simulink model "TosAeb"  (R2021a)
settings: Solver=FixedStepDiscrete  StartTime=0.0  StopTime=inf  FixedStep=0.01  RelTol=1e-3
undefined symbols (not defined in the file or in its folder): ACCModSts, AccSts1, AEB_ObjectMovingOrientation, AEB_ObjectMovingState, CN_BTCActive, CN_CurrentGearR, CN_CurrentGearR_DHT, CN_PTCActive, CN_VDCActive, CN_VSC_EspOff, CN_VSC_FCW_AEB_Error, CN_VSC_FCW_AEB_NoDispay, CN_VSC_FCW_AEB_PerfmDgdtn, CN_VSC_FCW_AEB_TempUnAvlbl, CN_VSC_VehStandstill, CNaccelAvoidMax, CNaccelAvoidMin, CNbrakePedalPress, CNcomfortStrLeftAccelMax, CNcomfortStrLeftAccelMin, CNcomfortStrRightAccelMax, CNcomfortStrRightAccelMin, CNDrvDoorOpen, CNDrvModeEco, CNDrvModeNorm, CNDrvModeOther, CNDrvModeSport, CNDrvSBRreminder, CNNull, CNobjInvalidID, CNPassDoorOpen, CNPassSBRreminder, CNPEBSAccelNegInf, CNPEBSAccelNull, CNPEBSTimeInf, CNPEBSTimeNull, CNSTOPMAX, CNSTOPNULL, CNsubjWidth, CNSwtOFF, CNSwtON, CNTTCMAX, CNTTCNULL, CNVehConfigAEBCycNone, CNVehConfigAEBNone, CNVehConfigAEBPedNone, CNVehConfigFCWNone, CNVehConfigJANone, CollisionSeverity, FALSE, FCWSnvty, JACycObjCalcInfoDefaultParams, JAPedObjCalcInfoDefaultParams, JAVehObjCalcInfoDefaultParams, latCycObjCalcInfoDefaultParams, latPedObjCalcInfoDefaultParams, longObjCalcInfoDefaultParams, ModSts, movingStatus, ObjectType ... (+1112)
libraries: simulink x3031, AEBSFunctionLib x50, Ta_Library x33, AEBSStateMachineLib x23, Mylibrary x12, simulink_extras x7, ObjSelect_lib x3, dspstat3 x2
(part 64 of 102)

== system TosAeb/FcwAebJa_Step_sys/ALG_AEBSystem/ALG_AEBController/AEB_LongTgtInfoCalc/LongObjCalcInfoCalc/AEBS_LongCollisionAssesApp/LatCollisionAvoidCalc/LatAvoidFlg4WarningJudge/accelLatAvoidThesMO/AccelLatAvoidThesCalcHmiLate ==
  subjVel : Inport
  Action Port : ActionPort  ActionPortLabel=default:
  accelLatAvoidThesLookupTable : Lookup_n-D  NumberOfTableDimensions=1  BreakpointsForDimension1=P_AEB_LongCA_LatAvoidAccelThesWarnHmiLateMOX  BeginIndexSearchUsingPreviousIndexResult=on  Table=P_AEB_LongCA_LatAvoidAccelThesWarnHmiLateMOY  ExtrapMethod=Clip  InternalRulePriority=Speed  RndMeth=Simplest  InputSameDT=on
  accelLatAvoidThes : Outport
  wires:
  subjVel/1 -> accelLatAvoidThesLookupTable/1
  accelLatAvoidThesLookupTable/1 -> accelLatAvoidThes/1

== system TosAeb/FcwAebJa_Step_sys/ALG_AEBSystem/ALG_AEBController/AEB_LongTgtInfoCalc/LongObjCalcInfoCalc/AEBS_LongCollisionAssesApp/LatCollisionAvoidCalc/LatAvoidFlg4WarningJudge/accelLatAvoidThesMO/AccelLatAvoidThesCalcHmiMid ==
  subjVel : Inport
  Action Port : ActionPort  ActionPortLabel=case [ HMISettingMiddle ]:
  accelLatAvoidThesLookupTable : Lookup_n-D  NumberOfTableDimensions=1  BreakpointsForDimension1=P_AEB_LongCA_LatAvoidAccelThesWarnHmiMidMOX  BeginIndexSearchUsingPreviousIndexResult=on  Table=P_AEB_LongCA_LatAvoidAccelThesWarnHmiMidMOY  ExtrapMethod=Clip  InternalRulePriority=Speed  RndMeth=Simplest  InputSameDT=on
  accelLatAvoidThes : Outport
  wires:
  subjVel/1 -> accelLatAvoidThesLookupTable/1
  accelLatAvoidThesLookupTable/1 -> accelLatAvoidThes/1

== system TosAeb/FcwAebJa_Step_sys/ALG_AEBSystem/ALG_AEBController/AEB_LongTgtInfoCalc/LongObjCalcInfoCalc/AEBS_LongCollisionAssesApp/LatCollisionAvoidCalc/LatAvoidFlg4WarningJudge/accelLatAvoidThesSO/AccelLatAvoidThesCalcHmiEarly ==
  subjVel : Inport
  Action Port : ActionPort  ActionPortLabel=case [ HMISettingEarly ]:
  accelLatAvoidThesLookupTable : Lookup_n-D  NumberOfTableDimensions=1  BreakpointsForDimension1=P_AEB_LongCA_LatAvoidAccelThesWarnHmiEarlySOX  BeginIndexSearchUsingPreviousIndexResult=on  Table=P_AEB_LongCA_LatAvoidAccelThesWarnHmiEarlySOY  ExtrapMethod=Clip  InternalRulePriority=Speed  RndMeth=Simplest  InputSameDT=on
  accelLatAvoidThes : Outport
  wires:
  accelLatAvoidThesLookupTable/1 -> accelLatAvoidThes/1
  subjVel/1 -> accelLatAvoidThesLookupTable/1

== system TosAeb/FcwAebJa_Step_sys/ALG_AEBSystem/ALG_AEBController/AEB_LongTgtInfoCalc/LongObjCalcInfoCalc/AEBS_LongCollisionAssesApp/LatCollisionAvoidCalc/LatAvoidFlg4WarningJudge/accelLatAvoidThesSO/AccelLatAvoidThesCalcHmiLate ==
  subjVel : Inport
  Action Port : ActionPort  ActionPortLabel=default:
  accelLatAvoidThesLookupTable : Lookup_n-D  NumberOfTableDimensions=1  BreakpointsForDimension1=P_AEB_LongCA_LatAvoidAccelThesWarnHmiLateSOX  BeginIndexSearchUsingPreviousIndexResult=on  Table=P_AEB_LongCA_LatAvoidAccelThesWarnHmiLateSOY  ExtrapMethod=Clip  InternalRulePriority=Speed  RndMeth=Simplest  InputSameDT=on
  accelLatAvoidThes : Outport
  wires:
  accelLatAvoidThesLookupTable/1 -> accelLatAvoidThes/1
  subjVel/1 -> accelLatAvoidThesLookupTable/1

== system TosAeb/FcwAebJa_Step_sys/ALG_AEBSystem/ALG_AEBController/AEB_LongTgtInfoCalc/LongObjCalcInfoCalc/AEBS_LongCollisionAssesApp/LatCollisionAvoidCalc/LatAvoidFlg4WarningJudge/accelLatAvoidThesSO/AccelLatAvoidThesCalcHmiMid ==
  subjVel : Inport
  Action Port : ActionPort  ActionPortLabel=case [ HMISettingMiddle ]:
  accelLatAvoidThesLookupTable : Lookup_n-D  NumberOfTableDimensions=1  BreakpointsForDimension1=P_AEB_LongCA_LatAvoidAccelThesWarnHmiMidSOX  BeginIndexSearchUsingPreviousIndexResult=on  Table=P_AEB_LongCA_LatAvoidAccelThesWarnHmiMidSOY  ExtrapMethod=Clip  InternalRulePriority=Speed  RndMeth=Simplest  InputSameDT=on
  accelLatAvoidThes : Outport
  wires:
  accelLatAvoidThesLookupTable/1 -> accelLatAvoidThes/1
  subjVel/1 -> accelLatAvoidThesLookupTable/1

== system TosAeb/FcwAebJa_Step_sys/ALG_AEBSystem/ALG_AEBController/AEB_LongTgtInfoCalc/LongObjCalcInfoCalc/AEBS_LongCollisionAssesApp/LongCollisionAvoidAssess/HighLvlBrkCalc/HighLvlBrkCalc4Brake/HighLvlBrkFlgJudge ==
  accelAvoidHighLvlBrk : Inport
  subjVel : Inport  Port=2
  Compare To Constant : simulink/Logic and Bit Operations/Compare To Constant  relop===  const=FALSE  OutDataTypeStr=boolean
  HighLvlBrkAccelAvoidThes4Brk : Lookup_n-D  NumberOfTableDimensions=1  BreakpointsForDimension1=P_AEB_LongCA_HighLvlBrkAccelAvoidThes4BrkX  Table=P_AEB_LongCA_HighLvlBrkAccelAvoidThes4BrkY  ExtrapMethod=Clip  InternalRulePriority=Speed  RndMeth=Simplest  InputSameDT=on
  P_LongCA_HighLvlBrkAccelAvoidThes : Constant  Commented=on  Value=P_AEB_LongCA_HighLvlBrkAccelAvoidThes4Warn
  Relational Operator : RelationalOperator  Operator=<=  OutDataTypeStr=boolean  RndMeth=Simplest
  highLvlBrkFlg : Outport
  wires:
  HighLvlBrkAccelAvoidThes4Brk/1 -> Relational Operator/2  "AEB_LongCA_HighLvlBrkAccelAvoidThes4Brk"
  accelAvoidHighLvlBrk/1 -> Relational Operator/1
  Relational Operator/1 -> Compare To Constant/1
  Compare To Constant/1 -> highLvlBrkFlg/1
  subjVel/1 -> HighLvlBrkAccelAvoidThes4Brk/1

== system TosAeb/FcwAebJa_Step_sys/ALG_AEBSystem/ALG_AEBController/AEB_LongTgtInfoCalc/LongObjCalcInfoCalc/AEBS_LongCollisionAssesApp/LongCollisionAvoidAssess/HighLvlBrkCalc/HighLvlBrkCalc4Brake/HighLvlBrkFlgJudge1 ==
  accelAvoidHighLvlBrk : Inport
  Logical Operator : Logic  Operator=NOT  AllPortsSameDT=off  OutDataTypeStr=boolean
  P_LongCA_HighLvlBrkAccelAvoidThes : Constant  Value=P_AEB_LongCA_HighLvlBrkAccelAvoidThes4Brake
  Relational Operator : RelationalOperator  Operator=<=  OutDataTypeStr=boolean  RndMeth=Simplest
  highLvlBrkFlg : Outport
  wires:
  P_LongCA_HighLvlBrkAccelAvoidThes/1 -> Relational Operator/2
  Relational Operator/1 -> Logical Operator/1
  accelAvoidHighLvlBrk/1 -> Relational Operator/1
  Logical Operator/1 -> highLvlBrkFlg/1

== system TosAeb/FcwAebJa_Step_sys/ALG_AEBSystem/ALG_AEBController/AEB_LongTgtInfoCalc/LongObjCalcInfoCalc/AEBS_LongCollisionAssesApp/LongCollisionAvoidAssess/HighLvlBrkCalc/HighLvlBrkCalc4Brake/accelAvoidHighLvlBrkSituation1 ==
  vx : Inport
  axAbsObj : Inport  Port=2
  vxAbsObj : Inport  Port=3
  subjVel : Inport  Port=4
  tObjStop : Inport  Port=5
  dxEgoBrake : Inport  Port=6
  function : TriggerPort  TriggerType=function-call  VariantControl=Variant
  accelAvoidLowLvlBrkCalc : Subsystem  TreatAsAtomicUnit=on
  accelAvoidLowLvlBrkCalc2 : Subsystem  Commented=on  TreatAsAtomicUnit=on
  accelAvoidHighLvlBrk : Outport
  wires:
  vx/1 -> accelAvoidLowLvlBrkCalc/1(vx)
  axAbsObj/1 -> accelAvoidLowLvlBrkCalc/2(axAbsObj)
  vxAbsObj/1 -> accelAvoidLowLvlBrkCalc/3(vxAbsObj)
  subjVel/1 -> accelAvoidLowLvlBrkCalc/4(subjVel)
  tObjStop/1 -> accelAvoidLowLvlBrkCalc/5(tObjStop)
  dxEgoBrake/1 -> accelAvoidLowLvlBrkCalc/6(dxEgoBrake)
  accelAvoidLowLvlBrkCalc/1(accelAvoidLowLvlBrk) -> accelAvoidHighLvlBrk/1

== system TosAeb/FcwAebJa_Step_sys/ALG_AEBSystem/ALG_AEBController/AEB_LongTgtInfoCalc/LongObjCalcInfoCalc/AEBS_LongCollisionAssesApp/LongCollisionAvoidAssess/HighLvlBrkCalc/HighLvlBrkCalc4Brake/accelAvoidHighLvlBrkSituation2 ==
  tReaction : Inport
  TTC : Inport  Port=2
  dxFrontBumper : Inport  Port=3
  vx : Inport  Port=4
  ax : Inport  Port=5
  subjLongAccel : Inport  Port=6
  subjVel : Inport  Port=7
  axAbsObj : Inport  Port=8
  function : TriggerPort  TriggerType=function-call  VariantControl=Variant
  CollisionJuge : Subsystem
  CollisonOcurred : Subsystem  TreatAsAtomicUnit=on
  If : If  IfExpression=u1
  Merge : Merge
  accelAvoidHighLvlBrkCalc : Subsystem  TreatAsAtomicUnit=on
  accelAvoidHighLvlBrk : Outport
  wires:
  tReaction/1 -> CollisionJuge/1(tReaction), accelAvoidHighLvlBrkCalc/3(tReaction)
  CollisionJuge/1(collisonFlg) -> If/1
  TTC/1 -> CollisionJuge/2(TTC)
  If/1 -> CollisonOcurred/Action
  If/2 -> accelAvoidHighLvlBrkCalc/Action
  vx/1 -> accelAvoidHighLvlBrkCalc/2(vx)
  ax/1 -> accelAvoidHighLvlBrkCalc/4(ax)
  subjLongAccel/1 -> accelAvoidHighLvlBrkCalc/5(subjLongAccel)
  subjVel/1 -> accelAvoidHighLvlBrkCalc/6(subjVel)
  axAbsObj/1 -> accelAvoidHighLvlBrkCalc/7(axAbsObj)
  CollisonOcurred/1(accelAvoidHighLvlBrk) -> Merge/1
  accelAvoidHighLvlBrkCalc/1(accelAvoidHighLvlBrk) -> Merge/2
  Merge/1 -> accelAvoidHighLvlBrk/1
  dxFrontBumper/1 -> accelAvoidHighLvlBrkCalc/1(dxFrontBumper)

== system TosAeb/FcwAebJa_Step_sys/ALG_AEBSystem/ALG_AEBController/AEB_LongTgtInfoCalc/LongObjCalcInfoCalc/AEBS_LongCollisionAssesApp/LongCollisionAvoidAssess/HighLvlBrkCalc/HighLvlBrkCalc4Brake/accelAvoidHighLvlBrkSituation3 ==
  note: if tRaction > TTC
      | 	CollisionOcurred
      | else
      | 	caculate AccelAvoid
  tReaction : Inport
  TTC : Inport  Port=2
  subjLongAccel : Inport  Port=3
  subjVel : Inport  Port=4
  axAbsObj : Inport  Port=5
  vxAbsObj : Inport  Port=6
  dxFrontBumper : Inport  Port=7
  function : TriggerPort  TriggerType=function-call  VariantControl=Variant
  CollisionJuge : Subsystem
  If : If  IfExpression=u1
  If Action Subsystem : Subsystem  TreatAsAtomicUnit=on
  If Action Subsystem1 : Subsystem  TreatAsAtomicUnit=on
  Merge : Merge
  accelAvoidHighLvlBrk : Outport
  wires:
  CollisionJuge/1(collisonFlg) -> If/1
  TTC/1 -> CollisionJuge/2(TTC)
  tReaction/1 -> CollisionJuge/1(tReaction), If Action Subsystem1/2(tReaction)
  If/1 -> If Action Subsystem/Action
  If/2 -> If Action Subsystem1/Action
  subjLongAccel/1 -> If Action Subsystem1/1(subjLongAccel)
  subjVel/1 -> If Action Subsystem1/3(subjVel)
  axAbsObj/1 -> If Action Subsystem1/4(axAbsObj)
  vxAbsObj/1 -> If Action Subsystem1/5(vxAbsObj)
  dxFrontBumper/1 -> If Action Subsystem1/6(dxFrontBumper)
  If Action Subsystem/1(accelAvoidHighLvlBrk) -> Merge/1
  If Action Subsystem1/1(accelAvoidHighLvlBrk) -> Merge/2
  Merge/1 -> accelAvoidHighLvlBrk/1

== system TosAeb/FcwAebJa_Step_sys/ALG_AEBSystem/ALG_AEBController/AEB_LongTgtInfoCalc/LongObjCalcInfoCalc/AEBS_LongCollisionAssesApp/LongCollisionAvoidAssess/HighLvlBrkCalc/HighLvlBrkCalc4Brake/accelAvoidHighLvlBrkSituation4 ==
  tReaction : Inport
  TTC : Inport  Port=2
  function : TriggerPort  TriggerType=function-call  VariantControl=Variant
  accelAvoidHighLvlBrkCalc : Subsystem
  accelAvoidHighLvlBrk : Outport
  wires:
  tReaction/1 -> accelAvoidHighLvlBrkCalc/1(tReaction)
  TTC/1 -> accelAvoidHighLvlBrkCalc/2(TTC)
  accelAvoidHighLvlBrkCalc/1(accelAvoidHighLvlBrk) -> accelAvoidHighLvlBrk/1

== system TosAeb/FcwAebJa_Step_sys/ALG_AEBSystem/ALG_AEBController/AEB_LongTgtInfoCalc/LongObjCalcInfoCalc/AEBS_LongCollisionAssesApp/LongCollisionAvoidAssess/HighLvlBrkCalc/HighLvlBrkCalc4Brake/accelAvoidHighLvlBrkSituation5 ==
  tEgoStop : Inport
  tObjStop : Inport  Port=2
  tReaction : Inport  Port=3
  TTC : Inport  Port=4
  subjLongAccel : Inport  Port=5
  subjVel : Inport  Port=6
  axAbsObj : Inport  Port=7
  dxFrontBumper : Inport  Port=8
  vxAbsObj : Inport  Port=9
  function : TriggerPort  TriggerType=function-call  VariantControl=Variant
  If : If  NumInputs=2  IfExpression=u1 <= u2
  If Action Subsystem : Subsystem  TreatAsAtomicUnit=on
  If Action Subsystem1 : Subsystem  TreatAsAtomicUnit=on
  Merge : Merge
  accelAvoidHighLvlBrk : Outport
  wires:
  [tEgoStop] -> If Action Subsystem1/4(tEgoStop)
  vxAbsObj/1 -> If Action Subsystem1/8(vxAbsObj)
  dxFrontBumper/1 -> If Action Subsystem1/7(dxFrontBumper)
  tEgoStop/1 -> If/1, [tEgoStop]
  tObjStop/1 -> If/2
  If/1 -> If Action Subsystem/Action
  If/2 -> If Action Subsystem1/Action
  If Action Subsystem/1(accelAvoidHighLvlBrk) -> Merge/1
  If Action Subsystem1/1(accelAvoidHighLvlBrk) -> Merge/2
  Merge/1 -> accelAvoidHighLvlBrk/1
  TTC/1 -> [TTC], If Action Subsystem1/2(TTC)
  tReaction/1 -> [tReaction], If Action Subsystem1/1(tReaction)
  subjLongAccel/1 -> If Action Subsystem1/3(subjLongAccel)
  subjVel/1 -> If Action Subsystem1/5(subjVel)
  axAbsObj/1 -> If Action Subsystem1/6(axAbsObj)
  [tReaction] -> If Action Subsystem/1(tReaction)
  [TTC] -> If Action Subsystem/2(TTC)

== system TosAeb/FcwAebJa_Step_sys/ALG_AEBSystem/ALG_AEBController/AEB_LongTgtInfoCalc/LongObjCalcInfoCalc/AEBS_LongCollisionAssesApp/LongCollisionAvoidAssess/HighLvlBrkCalc/HighLvlBrkCalc4Warning/HighLvlBrkFlgJudge ==
  accelAvoidHighLvlBrk : Inport
  subjVel : Inport  Port=2
  Compare To Constant : simulink/Logic and Bit Operations/Compare To Constant  relop===  const=FALSE  OutDataTypeStr=boolean
  HighLvlBrkAccelAvoidThes4Warn : Lookup_n-D  NumberOfTableDimensions=1  BreakpointsForDimension1=P_AEB_LongCA_HighLvlBrkAccelAvoidThes4WarnX  Table=P_AEB_LongCA_HighLvlBrkAccelAvoidThes4WarnY  ExtrapMethod=Clip  InternalRulePriority=Speed  RndMeth=Simplest  InputSameDT=on
  P_LongCA_HighLvlBrkAccelAvoidThes : Constant  Commented=on  Value=P_AEB_LongCA_HighLvlBrkAccelAvoidThes4Warn
  Relational Operator : RelationalOperator  Operator=<=  OutDataTypeStr=boolean  RndMeth=Simplest
  highLvlBrkFlg : Outport
  wires:
  HighLvlBrkAccelAvoidThes4Warn/1 -> Relational Operator/2  "AEB_LongCA_HighLvlBrkAccelAvoidThes4Warn"
  accelAvoidHighLvlBrk/1 -> Relational Operator/1
  Relational Operator/1 -> Compare To Constant/1
  Compare To Constant/1 -> highLvlBrkFlg/1
  subjVel/1 -> HighLvlBrkAccelAvoidThes4Warn/1

== system TosAeb/FcwAebJa_Step_sys/ALG_AEBSystem/ALG_AEBController/AEB_LongTgtInfoCalc/LongObjCalcInfoCalc/AEBS_LongCollisionAssesApp/LongCollisionAvoidAssess/HighLvlBrkCalc/HighLvlBrkCalc4Warning/accelAvoidHighLvlBrkSituation1 ==
  vx : Inport
  axAbsObj : Inport  Port=2
  vxAbsObj : Inport  Port=3
  subjVel : Inport  Port=4
  tObjStop : Inport  Port=5
  dxEgoBrake : Inport  Port=6
  function : TriggerPort  TriggerType=function-call  VariantControl=Variant
  accelAvoidLowLvlBrkCalc : Subsystem  TreatAsAtomicUnit=on
  accelAvoidLowLvlBrkCalc2 : Subsystem  Commented=on  TreatAsAtomicUnit=on
  accelAvoidHighLvlBrk : Outport
  wires:
  accelAvoidLowLvlBrkCalc/1(accelAvoidLowLvlBrk) -> accelAvoidHighLvlBrk/1
  dxEgoBrake/1 -> accelAvoidLowLvlBrkCalc/6(dxEgoBrake)
  tObjStop/1 -> accelAvoidLowLvlBrkCalc/5(tObjStop)
  subjVel/1 -> accelAvoidLowLvlBrkCalc/4(subjVel)
  vxAbsObj/1 -> accelAvoidLowLvlBrkCalc/3(vxAbsObj)
  axAbsObj/1 -> accelAvoidLowLvlBrkCalc/2(axAbsObj)
  vx/1 -> accelAvoidLowLvlBrkCalc/1(vx)

== system TosAeb/FcwAebJa_Step_sys/ALG_AEBSystem/ALG_AEBController/AEB_LongTgtInfoCalc/LongObjCalcInfoCalc/AEBS_LongCollisionAssesApp/LongCollisionAvoidAssess/HighLvlBrkCalc/HighLvlBrkCalc4Warning/accelAvoidHighLvlBrkSituation2 ==
  tReaction : Inport
  TTC : Inport  Port=2
  dxFrontBumper : Inport  Port=3
  vx : Inport  Port=4
  ax : Inport  Port=5
  subjLongAccel : Inport  Port=6
  subjVel : Inport  Port=7
  axAbsObj : Inport  Port=8
  function : TriggerPort  TriggerType=function-call  VariantControl=Variant
  CollisionJuge : Subsystem
  CollisonOcurred : Subsystem  TreatAsAtomicUnit=on
  If : If  IfExpression=u1
  Merge : Merge
  accelAvoidHighLvlBrkCalc : Subsystem  TreatAsAtomicUnit=on
  accelAvoidHighLvlBrk : Outport
  wires:
  dxFrontBumper/1 -> accelAvoidHighLvlBrkCalc/1(dxFrontBumper)
  Merge/1 -> accelAvoidHighLvlBrk/1
  accelAvoidHighLvlBrkCalc/1(accelAvoidHighLvlBrk) -> Merge/2
  CollisonOcurred/1(accelAvoidHighLvlBrk) -> Merge/1
  axAbsObj/1 -> accelAvoidHighLvlBrkCalc/7(axAbsObj)
  subjVel/1 -> accelAvoidHighLvlBrkCalc/6(subjVel)
  subjLongAccel/1 -> accelAvoidHighLvlBrkCalc/5(subjLongAccel)
  ax/1 -> accelAvoidHighLvlBrkCalc/4(ax)
  vx/1 -> accelAvoidHighLvlBrkCalc/2(vx)
  If/2 -> accelAvoidHighLvlBrkCalc/Action
  If/1 -> CollisonOcurred/Action
  TTC/1 -> CollisionJuge/2(TTC)
  CollisionJuge/1(collisonFlg) -> If/1
  tReaction/1 -> accelAvoidHighLvlBrkCalc/3(tReaction), CollisionJuge/1(tReaction)

== system TosAeb/FcwAebJa_Step_sys/ALG_AEBSystem/ALG_AEBController/AEB_LongTgtInfoCalc/LongObjCalcInfoCalc/AEBS_LongCollisionAssesApp/LongCollisionAvoidAssess/HighLvlBrkCalc/HighLvlBrkCalc4Warning/accelAvoidHighLvlBrkSituation3 ==
  note: if tRaction > TTC
      | 	CollisionOcurred
      | else
      | 	caculate AccelAvoid
  tReaction : Inport
  TTC : Inport  Port=2
  subjLongAccel : Inport  Port=3
  subjVel : Inport  Port=4
  axAbsObj : Inport  Port=5
  vxAbsObj : Inport  Port=6
  dxFrontBumper : Inport  Port=7
  function : TriggerPort  TriggerType=function-call  VariantControl=Variant
  CollisionJuge : Subsystem  TreatAsAtomicUnit=on
  If : If  IfExpression=u1
  If Action Subsystem : Subsystem  TreatAsAtomicUnit=on
  If Action Subsystem1 : Subsystem  TreatAsAtomicUnit=on
  Merge : Merge
  accelAvoidHighLvlBrk : Outport
  wires:
  Merge/1 -> accelAvoidHighLvlBrk/1
  If Action Subsystem1/1(accelAvoidHighLvlBrk) -> Merge/2
  If Action Subsystem/1(accelAvoidHighLvlBrk) -> Merge/1
  dxFrontBumper/1 -> If Action Subsystem1/6(dxFrontBumper)
  vxAbsObj/1 -> If Action Subsystem1/5(vxAbsObj)
  axAbsObj/1 -> If Action Subsystem1/4(axAbsObj)
  subjVel/1 -> If Action Subsystem1/3(subjVel)
  subjLongAccel/1 -> If Action Subsystem1/1(subjLongAccel)
  If/2 -> If Action Subsystem1/Action
  If/1 -> If Action Subsystem/Action
  tReaction/1 -> If Action Subsystem1/2(tReaction), CollisionJuge/1(tReaction)
  TTC/1 -> CollisionJuge/2(TTC)
  CollisionJuge/1(collisonFlg) -> If/1

== system TosAeb/FcwAebJa_Step_sys/ALG_AEBSystem/ALG_AEBController/AEB_LongTgtInfoCalc/LongObjCalcInfoCalc/AEBS_LongCollisionAssesApp/LongCollisionAvoidAssess/HighLvlBrkCalc/HighLvlBrkCalc4Warning/accelAvoidHighLvlBrkSituation4 ==
  tReaction : Inport
  TTC : Inport  Port=2
  function : TriggerPort  TriggerType=function-call  VariantControl=Variant
  accelAvoidHighLvlBrkCalc : Subsystem
  accelAvoidHighLvlBrk : Outport
  wires:
  accelAvoidHighLvlBrkCalc/1(accelAvoidHighLvlBrk) -> accelAvoidHighLvlBrk/1
  TTC/1 -> accelAvoidHighLvlBrkCalc/2(TTC)
  tReaction/1 -> accelAvoidHighLvlBrkCalc/1(tReaction)

== system TosAeb/FcwAebJa_Step_sys/ALG_AEBSystem/ALG_AEBController/AEB_LongTgtInfoCalc/LongObjCalcInfoCalc/AEBS_LongCollisionAssesApp/LongCollisionAvoidAssess/HighLvlBrkCalc/HighLvlBrkCalc4Warning/accelAvoidHighLvlBrkSituation5 ==
  tEgoStop : Inport
  tObjStop : Inport  Port=2
  tReaction : Inport  Port=3
  TTC : Inport  Port=4
  subjLongAccel : Inport  Port=5
  subjVel : Inport  Port=6
  axAbsObj : Inport  Port=7
  dxFrontBumper : Inport  Port=8
  vxAbsObj : Inport  Port=9
  function : TriggerPort  TriggerType=function-call  VariantControl=Variant
  If : If  NumInputs=2  IfExpression=u1 <= u2
  If Action Subsystem : Subsystem  TreatAsAtomicUnit=on
  If Action Subsystem1 : Subsystem  TreatAsAtomicUnit=on
  Merge : Merge
  accelAvoidHighLvlBrk : Outport
  wires:
  [TTC] -> If Action Subsystem/2(TTC)
  [tReaction] -> If Action Subsystem/1(tReaction)
  axAbsObj/1 -> If Action Subsystem1/6(axAbsObj)
  subjVel/1 -> If Action Subsystem1/5(subjVel)
  subjLongAccel/1 -> If Action Subsystem1/3(subjLongAccel)
  tReaction/1 -> If Action Subsystem1/1(tReaction), [tReaction]
  TTC/1 -> If Action Subsystem1/2(TTC), [TTC]
  Merge/1 -> accelAvoidHighLvlBrk/1
  If Action Subsystem1/1(accelAvoidHighLvlBrk) -> Merge/2
  If Action Subsystem/1(accelAvoidHighLvlBrk) -> Merge/1
  If/2 -> If Action Subsystem1/Action
  If/1 -> If Action Subsystem/Action
  tObjStop/1 -> If/2
  tEgoStop/1 -> [tEgoStop], If/1
  dxFrontBumper/1 -> If Action Subsystem1/7(dxFrontBumper)
  vxAbsObj/1 -> If Action Subsystem1/8(vxAbsObj)
  [tEgoStop] -> If Action Subsystem1/4(tEgoStop)

== system TosAeb/FcwAebJa_Step_sys/ALG_AEBSystem/ALG_AEBController/AEB_LongTgtInfoCalc/LongObjCalcInfoCalc/AEBS_LongCollisionAssesApp/LongCollisionAvoidAssess/LowLvlBrkCalc/LowLvlBrkFlg4WarningJudge/LowLvlBrkAccelThesCalcHMIEarly ==
  subjVel : Inport
  Action Port : ActionPort  ActionPortLabel=case [ HMISettingEarly ]:
  1-D Lookup Table : Lookup_n-D  NumberOfTableDimensions=1  BreakpointsForDimension1=P_AEB_LongCA_LowLvlBrkAccelAvoidThesWarnHmiEarlyX  BeginIndexSearchUsingPreviousIndexResult=on  Table=P_AEB_LongCA_LowLvlBrkAccelAvoidThesWarnHmiEarlyY  ExtrapMethod=Clip  InternalRulePriority=Speed  RndMeth=Simplest  InputSameDT=on
  LowLvlBrkAccelThes : Outport
  wires:
  1-D Lookup Table/1 -> LowLvlBrkAccelThes/1
  subjVel/1 -> 1-D Lookup Table/1

== system TosAeb/FcwAebJa_Step_sys/ALG_AEBSystem/ALG_AEBController/AEB_LongTgtInfoCalc/LongObjCalcInfoCalc/AEBS_LongCollisionAssesApp/LongCollisionAvoidAssess/LowLvlBrkCalc/LowLvlBrkFlg4WarningJudge/LowLvlBrkAccelThesCalcHMILate ==
  subjVel : Inport
  Action Port : ActionPort  ActionPortLabel=default:
  1-D Lookup Table2 : Lookup_n-D  NumberOfTableDimensions=1  BreakpointsForDimension1=P_AEB_LongCA_LowLvlBrkAccelAvoidThesWarnHmiLateX  BeginIndexSearchUsingPreviousIndexResult=on  Table=P_AEB_LongCA_LowLvlBrkAccelAvoidThesWarnHmiLateY  ExtrapMethod=Clip  InternalRulePriority=Speed  RndMeth=Simplest  InputSameDT=on
  LowLvlBrkAccelThes : Outport
  wires:
  1-D Lookup Table2/1 -> LowLvlBrkAccelThes/1
  subjVel/1 -> 1-D Lookup Table2/1

== system TosAeb/FcwAebJa_Step_sys/ALG_AEBSystem/ALG_AEBController/AEB_LongTgtInfoCalc/LongObjCalcInfoCalc/AEBS_LongCollisionAssesApp/LongCollisionAvoidAssess/LowLvlBrkCalc/LowLvlBrkFlg4WarningJudge/LowLvlBrkAccelThesCalcHMIMiddle ==
  subjVel : Inport
  Action Port : ActionPort  ActionPortLabel=case [ HMISettingMiddle ]:
  1-D Lookup Table1 : Lookup_n-D  NumberOfTableDimensions=1  BreakpointsForDimension1=P_AEB_LongCA_LowLvlBrkAccelAvoidThesWarnHmiMidX  BeginIndexSearchUsingPreviousIndexResult=on  Table=P_AEB_LongCA_LowLvlBrkAccelAvoidThesWarnHmiMidY  ExtrapMethod=Clip  InternalRulePriority=Speed  RndMeth=Simplest  InputSameDT=on
  LowLvlBrkAccelThes : Outport
  wires:
  1-D Lookup Table1/1 -> LowLvlBrkAccelThes/1
  subjVel/1 -> 1-D Lookup Table1/1

== system TosAeb/FcwAebJa_Step_sys/ALG_AEBSystem/ALG_AEBController/AEB_LongTgtInfoCalc/LongObjCalcInfoCalc/AEBS_LongCollisionAssesApp/LongCollisionAvoidAssess/LowLvlBrkCalc/accelAvoidLowLvlBrkCalc/Situation1 ==
  dxEgoBrake : Inport
  vx : Inport  Port=2
  tObjStop : Inport  Port=3
  subjVel : Inport  Port=4
  vxAbsObj : Inport  Port=5
  axAbsObj : Inport  Port=6
  function : TriggerPort  TriggerType=function-call  VariantControl=Variant
  Bus Creator : BusCreator  Inputs=6
  Bus Selector : BusSelector  OutputSignals=dxEgoBrake,vx,tObjStop
  Bus Selector1 : BusSelector  OutputSignals=axAbsObj,vx,dxEgoBrake
  Bus Selector2 : BusSelector  OutputSignals=subjVel,vxAbsObj,axAbsObj,dxEgoBrake
  Case1 : Subsystem  TreatAsAtomicUnit=on
  Case2 : Subsystem  TreatAsAtomicUnit=on
  If : If  IfExpression=u1
  Merge : Merge
  collisionFirstFlgCalc : Subsystem
  accelAvoidLowLvlBrk : Outport
  wires:
  collisionFirstFlgCalc/1(collisionFirstFlg) -> If/1
  Bus Selector/3 -> collisionFirstFlgCalc/3(tObjStop)  "<tObjStop>"
  Bus Selector/2 -> collisionFirstFlgCalc/2(vx)  "<vx>"
  Bus Selector/1 -> collisionFirstFlgCalc/1(dxEgoBrake)  "<dxEgoBrake>"
  Bus Selector2/4 -> Case2/4(dxEgoBrake)  "<dxEgoBrake>"
  Bus Selector2/3 -> Case2/3(axAbsObj)  "<axAbsObj>"
  Bus Selector2/2 -> Case2/2(vxAbsObj)  "<vxAbsObj>"
  Bus Selector2/1 -> Case2/1(subjVel)  "<subjVel>"
  Bus Selector1/3 -> Case1/3(dxEgoBrake)  "<dxEgoBrake>"
  Bus Selector1/2 -> Case1/2(vx)  "<vx>"
  Bus Selector1/1 -> Case1/1(axAbsObj)  "<axAbsObj>"
  Bus Creator/1 -> Bus Selector/1, Bus Selector1/1, Bus Selector2/1
  vxAbsObj/1 -> Bus Creator/5  "vxAbsObj"
  axAbsObj/1 -> Bus Creator/6  "axAbsObj"
  dxEgoBrake/1 -> Bus Creator/1  "dxEgoBrake"
  subjVel/1 -> Bus Creator/4  "subjVel"
  vx/1 -> Bus Creator/2  "vx"
  tObjStop/1 -> Bus Creator/3  "tObjStop"
  [CASE2] -> Case2/Action
  If/2 -> [CASE2]
  Case2/1(accelAvoid) -> Merge/2
  Case1/1(accelAvoid) -> Merge/1
  Merge/1 -> accelAvoidLowLvlBrk/1
  If/1 -> Case1/Action

== system TosAeb/FcwAebJa_Step_sys/ALG_AEBSystem/ALG_AEBController/AEB_LongTgtInfoCalc/LongObjCalcInfoCalc/AEBS_LongCollisionAssesApp/LongCollisionAvoidAssess/LowLvlBrkCalc/accelAvoidLowLvlBrkCalc/Situation2 ==
  axAbsObj : Inport
  vx : Inport  Port=2
  dxEgoBrake : Inport  Port=3
  function : TriggerPort  TriggerType=function-call  VariantControl=Variant
  Divide : Product  Inputs=*/
  Gain : Gain  Gain=2
  Math Function : Math  Operator=square  SignedPower=on  SaturateOnIntegerOverflow=on
  Subtract : Sum  Inputs=+-
  accelAvoidLowLvlBrk : Outport
  wires:
  Divide/1 -> Subtract/2
  Subtract/1 -> accelAvoidLowLvlBrk/1
  Gain/1 -> Divide/2
  dxEgoBrake/1 -> Gain/1
  Math Function/1 -> Divide/1
  vx/1 -> Math Function/1
  axAbsObj/1 -> Subtract/1

== system TosAeb/FcwAebJa_Step_sys/ALG_AEBSystem/ALG_AEBController/AEB_LongTgtInfoCalc/LongObjCalcInfoCalc/AEBS_LongCollisionAssesApp/LongCollisionAvoidAssess/LowLvlBrkCalc/accelAvoidLowLvlBrkCalc/Situation3 ==
  note: aEgo^2
      | aAvoid = ----------------------------------
      | 		vObj^2
      | 		---------  - 2(dRel - dScure)
      | 		aObj
  subjVel : Inport
  vxAbsObj : Inport  Port=2
  axAbsObj : Inport  Port=3
  dxEgoBrake : Inport  Port=4
  function : TriggerPort  TriggerType=function-call  VariantControl=Variant
  Divide : Product  Inputs=*/
  Divide1 : Product  Inputs=*/
  Gain : Gain  Gain=2
  Math Function : Math  Operator=square  SignedPower=on  SaturateOnIntegerOverflow=on
  Math Function1 : Math  Operator=square  SignedPower=on  SaturateOnIntegerOverflow=on
  Subtract : Sum  Inputs=+-
  accelAvoidLowLvlBrk : Outport
  wires:
  Subtract/1 -> Divide1/2
  dxEgoBrake/1 -> Gain/1
  axAbsObj/1 -> Divide/2
  Divide/1 -> Subtract/1
  Math Function1/1 -> Divide1/1
  Divide1/1 -> accelAvoidLowLvlBrk/1
  Gain/1 -> Subtract/2
  Math Function/1 -> Divide/1
  subjVel/1 -> Math Function1/1
  vxAbsObj/1 -> Math Function/1

== system TosAeb/FcwAebJa_Step_sys/ALG_AEBSystem/ALG_AEBController/AEB_LongTgtInfoCalc/LongObjCalcInfoCalc/AEBS_LongCollisionAssesApp/LongCollisionAvoidAssess/LowLvlBrkCalc/accelAvoidLowLvlBrkCalc/Situation4 ==
  function : TriggerPort  TriggerType=function-call  VariantControl=Variant
  Constant : Constant  Value=CNNull  VectorParams1D=off
  accelAvoidLowLvlBrk : Outport
  wires:
  Constant/1 -> accelAvoidLowLvlBrk/1

== system TosAeb/FcwAebJa_Step_sys/ALG_AEBSystem/ALG_AEBController/AEB_LongTgtInfoCalc/LongObjCalcInfoCalc/AEBS_LongCollisionAssesApp/LongCollisionAvoidAssess/LowLvlBrkCalc/accelAvoidLowLvlBrkCalc/accelAvoidLowLvlBrkLimit ==
  accelAvoidLowLvlBrkNoLimit : Inport
  Saturation : Saturate  UpperLimit=CNNull  LowerLimit=CNaccelAvoidMin
      settings: AttributesFormatString=|
      | UpperLimit:%<UpperLimit>
      | LowerLimit:%<LowerLimit>
  accelAvoidLowLvlBrk : Outport
  wires:
  Saturation/1 -> accelAvoidLowLvlBrk/1
  accelAvoidLowLvlBrkNoLimit/1 -> Saturation/1

== system TosAeb/FcwAebJa_Step_sys/ALG_AEBSystem/ALG_AEBController/AEB_LongTgtInfoCalc/LongObjCalcInfoCalc/AEBS_LongCollisionAssesApp/TTCCalc/TTCCalc/Situation2/SloveTTCQuadraticEquation ==
  accelRel : Inport
  velRel : Inport  Port=2
  distRel : Inport  Port=3
  AccelRelEqualsZero : Subsystem  TreatAsAtomicUnit=on
  AccelRelUnequalZero : Subsystem  TreatAsAtomicUnit=on
  If1 : If  IfExpression=u1
  Merge : Merge
  accelZeroFlgCalc : Subsystem
  TTC : Outport
  wires:
  If1/2 -> AccelRelUnequalZero/Action
  If1/1 -> AccelRelEqualsZero/Action
  AccelRelEqualsZero/1(TTC) -> Merge/1  "TTC"
  AccelRelUnequalZero/1(TTC) -> Merge/2  "TTC"
  Merge/1 -> TTC/1  "TTC"
  accelRel/1 -> AccelRelUnequalZero/1(accelRel), accelZeroFlgCalc/1(accel)  "accelRel"
  velRel/1 -> [velRel], AccelRelUnequalZero/2(velRel)  "velRel"
  distRel/1 -> [distRel], AccelRelUnequalZero/3(distRel)  "distRel"
  [velRel] -> AccelRelEqualsZero/2(velRel)
  [distRel] -> AccelRelEqualsZero/1(distRel)
  accelZeroFlgCalc/1(accelZeroFlg) -> If1/1

== system TosAeb/FcwAebJa_Step_sys/ALG_AEBSystem/ALG_AEBController/AEB_LongTgtInfoCalc/LongObjCalcInfoCalc/AEBS_LongCollisionAssesApp/TTCCalc/TTCCalc/Situation2/TTCArbitrate ==
  tempTTC : Inport
  tEgoStop : Inport  Port=2
  tObjStop : Inport  Port=3
  Constant : Constant  Value=CNTTCMAX
  Logical Operator : Logic  AllPortsSameDT=off  OutDataTypeStr=boolean
  Relational Operator : RelationalOperator  OutDataTypeStr=boolean  RndMeth=Simplest
  Relational Operator1 : RelationalOperator  Operator=<=  OutDataTypeStr=boolean  RndMeth=Simplest
  Switch : Switch  Criteria=u2 ~= 0
  TTC : Outport
  wires:
  Switch/1 -> TTC/1
  tempTTC/1 -> Switch/1, Relational Operator/1, Relational Operator1/1
  Relational Operator/1 -> Logical Operator/1
  Relational Operator1/1 -> Logical Operator/2
  Logical Operator/1 -> Switch/2
  tEgoStop/1 -> Relational Operator/2
  tObjStop/1 -> Relational Operator1/2
  Constant/1 -> Switch/3

== system TosAeb/FcwAebJa_Step_sys/ALG_AEBSystem/ALG_AEBController/AEB_LongTgtInfoCalc/LongObjCalcInfoCalc/AEBS_LongCollisionAssesApp/TTCCalc/TTCCalc/Situation2/distCorrCalc ==
  note: disCorr = distEgo + dxTargetObjCalc
  note: if Abs(subjLongAccel) < 0.1
      | disEgo = 0
      | else
      | subjVel^2
      | disEgo = ------------------------
      | 2* subjLongAccel
  subjLongAccel : Inport
  subjVel : Inport  Port=2
  dxFrontBumper : Inport  Port=3
  AccelEqualZeroJudge : Subsystem
  DistEgoJudge : If  IfExpression=u1
  Merge : Merge
  distCorrCalc : Subsystem
  subjLongAccelEqualZero : Subsystem  TreatAsAtomicUnit=on
  subjLongAccelNoEqualZero : Subsystem  TreatAsAtomicUnit=on
  distCorr : Outport
  wires:
  DistEgoJudge/2 -> subjLongAccelNoEqualZero/Action
  DistEgoJudge/1 -> subjLongAccelEqualZero/Action
  subjLongAccelEqualZero/1(distEgo) -> Merge/1
  subjLongAccelNoEqualZero/1(distEgo) -> Merge/2
  subjVel/1 -> subjLongAccelNoEqualZero/1(subjVel)
  subjLongAccel/1 -> AccelEqualZeroJudge/1(subjLongAccel), subjLongAccelNoEqualZero/2(subjLongAccel)
  Merge/1 -> distCorrCalc/1(distEgo)
  dxFrontBumper/1 -> distCorrCalc/2(dxFrontBumper)
  distCorrCalc/1(distCorr) -> distCorr/1
  AccelEqualZeroJudge/1(accelEqualZeroFlg) -> DistEgoJudge/1

== system TosAeb/FcwAebJa_Step_sys/ALG_AEBSystem/ALG_AEBController/AEB_LongTgtInfoCalc/LongObjCalcInfoCalc/AEBS_LongCollisionAssesApp/TTCCalc/TTCCalc/Situation3/SloveTTCQuadraticEquation ==
  accelRel : Inport
  velRel : Inport  Port=2
  distRel : Inport  Port=3
  AccelRelEqualsZero : Subsystem  TreatAsAtomicUnit=on
  AccelRelUnequalZero : Subsystem  TreatAsAtomicUnit=on
  If1 : If  IfExpression=u1
  Merge : Merge
  accelZeroFlgCalc : Subsystem
  TTC : Outport
  wires:
  If1/2 -> AccelRelUnequalZero/Action
  If1/1 -> AccelRelEqualsZero/Action
  AccelRelEqualsZero/1(TTC) -> Merge/1  "TTC"
  AccelRelUnequalZero/1(TTC) -> Merge/2  "TTC"
  Merge/1 -> TTC/1  "TTC"
  accelRel/1 -> AccelRelUnequalZero/1(accelRel), accelZeroFlgCalc/1(accel)  "accelRel"
  velRel/1 -> [velRel], AccelRelUnequalZero/2(velRel)  "velRel"
  distRel/1 -> [distRel], AccelRelUnequalZero/3(distRel)  "distRel"
  [velRel] -> AccelRelEqualsZero/2(velRel)
  [distRel] -> AccelRelEqualsZero/1(distRel)
  accelZeroFlgCalc/1(accelZeroFlg) -> If1/1

== system TosAeb/FcwAebJa_Step_sys/ALG_AEBSystem/ALG_AEBController/AEB_LongTgtInfoCalc/LongObjCalcInfoCalc/AEBS_LongCollisionAssesApp/TTCCalc/TTCCalc/Situation3/TTCArbitute ==
  tempTTC : Inport
  tEgoStop : Inport  Port=2
  tObjStop : Inport  Port=3
  Constant : Constant  Value=CNTTCMAX
  Logical Operator : Logic  AllPortsSameDT=off  OutDataTypeStr=boolean
  Relational Operator : RelationalOperator  Operator=<=  OutDataTypeStr=boolean  RndMeth=Simplest
  Relational Operator1 : RelationalOperator  OutDataTypeStr=boolean  RndMeth=Simplest
  Switch : Switch  Criteria=u2 > Threshold
  TTC : Outport
  wires:
  Constant/1 -> Switch/3
  tObjStop/1 -> Relational Operator1/2
  tEgoStop/1 -> Relational Operator/2
  Logical Operator/1 -> Switch/2
  Relational Operator1/1 -> Logical Operator/2
  Relational Operator/1 -> Logical Operator/1
  tempTTC/1 -> Relational Operator1/1, Relational Operator/1, Switch/1
  Switch/1 -> TTC/1

== system TosAeb/FcwAebJa_Step_sys/ALG_AEBSystem/ALG_AEBController/AEB_LongTgtInfoCalc/LongObjCalcInfoCalc/AEBS_LongCollisionAssesApp/TTCCalc/TTCCalc/Situation3/distCorrCalc ==
  note: disCorr = dxTargetObjCalc - distObj
  note: if abs(subjLongAccel) < 0.1
      | disObj = 0
      | else
      | vxAbsTargetObjCalc^2
      | disObj = ------------------------------------
      | 2* axAbsTargetObjCalc
  axAbsObj : Inport
  vxAbsObj : Inport  Port=2
  dxFrontBumper : Inport  Port=3
  AccelZeroJudge : Subsystem
  AxObjEqualZero : Subsystem  TreatAsAtomicUnit=on
  AxObjUnequalZero : Subsystem  TreatAsAtomicUnit=on
  If : If  IfExpression=u1
  Merge : Merge
  distCorrCalc : Subsystem
  distCorr : Outport
  wires:
  Merge/1 -> distCorrCalc/2(distObj)
  axAbsObj/1 -> AccelZeroJudge/1(axAbsObj), AxObjUnequalZero/2(axAbsObj)
  vxAbsObj/1 -> AxObjUnequalZero/1(vxAbsObj)
  AxObjUnequalZero/1(distObj) -> Merge/2
  AxObjEqualZero/1(distObj) -> Merge/1
  If/1 -> AxObjEqualZero/Action
  If/2 -> AxObjUnequalZero/Action
  dxFrontBumper/1 -> distCorrCalc/1(dxFrontBumper)
  distCorrCalc/1(distCorr) -> distCorr/1
  AccelZeroJudge/1(accelZeroFlg) -> If/1

== system TosAeb/FcwAebJa_Step_sys/ALG_AEBSystem/ALG_AEBController/AEB_LongTgtInfoCalc/LongObjCalcInfoCalc/AEBS_LongCollisionAssesApp/TTCCalc/TTCCalc/Situation3/subjAccelVelTrans ==
  subjAccel : Inport
  subjVel : Inport  Port=2
  Gain : Gain  Gain=-1
  Gain1 : Gain  Gain=-1
  subjAccelNeg : Outport
  subjVelNeg : Outport  Port=2
  wires:
  subjAccel/1 -> Gain/1
  subjVel/1 -> Gain1/1
  Gain/1 -> subjAccelNeg/1
  Gain1/1 -> subjVelNeg/1

== system TosAeb/FcwAebJa_Step_sys/ALG_AEBSystem/ALG_AEBController/AEB_LongTgtInfoCalc/LongObjCalcInfoCalc/AEBS_LongCollisionAssesApp/TTCCalc/TTCCalc/SloveTTCQuadraticEquation/AccelRelEqualsZero ==
  distRel : Inport
  velRel : Inport  Port=2
  AccelRelEqualZero : ActionPort  ActionPortLabel=if(u1)
  Chart : Stateflow chart  TreatAsAtomicUnit=on
  TTC : Outport
  wires:
  Chart/1(TTC) -> TTC/1  "TTC"
  distRel/1 -> Chart/1(distRel)  "distRel"
  velRel/1 -> Chart/2(velRel)  "velRel"

== system TosAeb/FcwAebJa_Step_sys/ALG_AEBSystem/ALG_AEBController/AEB_LongTgtInfoCalc/LongObjCalcInfoCalc/AEBS_LongCollisionAssesApp/TTCCalc/TTCCalc/SloveTTCQuadraticEquation/AccelRelUnequalZero ==
  accelRel : Inport
  velRel : Inport  Port=2
  distRel : Inport  Port=3
  AccelRelUnequalZero : ActionPort  ActionPortLabel=else
  DeltaCalcu : Subsystem
  DeltaGreaterEqualZero : Subsystem  TreatAsAtomicUnit=on
  DeltaSmallerZero : Subsystem  TreatAsAtomicUnit=on
  JudeDelta : If  IfExpression=u1 >= 0
  Merge : Merge
  TTC : Outport
  wires:
  Merge/1 -> TTC/1  "TTC"
  DeltaCalcu/1(delta) -> JudeDelta/1, DeltaGreaterEqualZero/1(delta)  "delta"
  accelRel/1 -> DeltaCalcu/1(accelRel), DeltaGreaterEqualZero/3(accelRel)  "accelRel"
  velRel/1 -> DeltaCalcu/2(velRel), DeltaGreaterEqualZero/2(velRel)  "velRel"
  distRel/1 -> DeltaCalcu/3(distRel)  "distRel"
  DeltaSmallerZero/1(TTC) -> Merge/2
  DeltaGreaterEqualZero/1(TTC) -> Merge/1
  JudeDelta/1 -> DeltaGreaterEqualZero/Action
  JudeDelta/2 -> [DeltaSmallZero]
  [DeltaSmallZero] -> DeltaSmallerZero/Action

== system TosAeb/FcwAebJa_Step_sys/ALG_AEBSystem/ALG_AEBController/AEB_LongTgtInfoCalc/LongObjCalcInfoCalc/AEBS_LongCollisionAssesApp/TTCCalc/TTCCalc/SloveTTCQuadraticEquation/accelZeroFlgCalc ==
  accel : Inport
  Abs : Abs
  P_LongCA_AccelObjFiltThesTTC : simulink/Logic and Bit Operations/Compare To Constant  relop=<=  const=P_AEB_LongCA_AccelObjFiltThesTTC  OutDataTypeStr=boolean
  accelZeroFlg : Outport
  wires:
  Abs/1 -> P_LongCA_AccelObjFiltThesTTC/1
  P_LongCA_AccelObjFiltThesTTC/1 -> accelZeroFlg/1  "accelZeroFlg"
  accel/1 -> Abs/1  "accel"

== system TosAeb/FcwAebJa_Step_sys/ALG_AEBSystem/ALG_AEBController/AEB_LongTgtInfoCalc/LongObjCalcInfoCalc/AEBS_LongCollisionAssesApp/TTCCalc/TTCCalc/TimegapCalc/If Action Subsystem ==
  Action Port : ActionPort  ActionPortLabel=if(u1)
  Constant : Constant  Value=CNTTCMAX
  TTC : Outport
  wires:
  Constant/1 -> TTC/1

== system TosAeb/FcwAebJa_Step_sys/ALG_AEBSystem/ALG_AEBController/AEB_LongTgtInfoCalc/LongObjCalcInfoCalc/AEBS_LongCollisionAssesApp/TTCCalc/TTCCalc/TimegapCalc/If Action Subsystem1 ==
  dxFrontBumper : Inport
  vx : Inport  Port=2
  Action Port : ActionPort  ActionPortLabel=else
  Abs : Abs
  Abs1 : Abs
  Divide : Product  Inputs=*/
  TTC : Outport
  wires:
  vx/1 -> Abs1/1
  dxFrontBumper/1 -> Abs/1
  Divide/1 -> TTC/1
  Abs/1 -> Divide/1
  Abs1/1 -> Divide/2

== system TosAeb/FcwAebJa_Step_sys/ALG_AEBSystem/ALG_AEBController/AEB_LongTgtInfoCalc/LongObjCalcInfoCalc/AEBS_LongCollisionAssesApp/TTCCalc/TTCCalc/TimegapCalc/subjVelZeroFlgCalc ==
  vx : Inport
  Abs : Abs
  Compare To Constant : simulink/Logic and Bit Operations/Compare To Constant  relop=<=  const=0.1  OutDataTypeStr=boolean
  subjVelZeroFlg : Outport
  wires:
  Compare To Constant/1 -> subjVelZeroFlg/1
  vx/1 -> Abs/1
  Abs/1 -> Compare To Constant/1

== system TosAeb/FcwAebJa_Step_sys/ALG_AEBSystem/ALG_AEBController/AEB_LongTgtInfoCalc/LongObjCalcInfoCalc/AEBS_LongCollisionAssesApp/TTCCalc/TTCCalc/tEgoStopCalc/StopTimeFlow/tStopFuncCalc ==
  velRel : Inport
  accelRel : Inport  Port=2
  tStopFuncCalc : TriggerPort  TriggerType=function-call  VariantControl=Variant
      settings: CopyFcn=Stateflow.SLINSF.SimulinkMan.removeBlockPreDeleteFcn  PreDeleteFcn=Stateflow.SLINSF.SimulinkMan.unsafePredeleteFcn
  Abs : Abs
  Abs1 : Abs
  Divide : Product  Inputs=*/
  temptStop : Outport
  wires:
  velRel/1 -> Abs/1
  Abs/1 -> Divide/1
  Abs1/1 -> Divide/2
  Divide/1 -> temptStop/1
  accelRel/1 -> Abs1/1

== system TosAeb/FcwAebJa_Step_sys/ALG_AEBSystem/ALG_AEBController/AEB_LongTgtInfoCalc/LongObjCalcInfoCalc/AEBS_LongCollisionAssesApp/TTCCalc/TTCCalc/tObjStopCalc/StopTimeFlow/tStopFuncCalc ==
  velRel : Inport
  accelRel : Inport  Port=2
  tStopFuncCalc : TriggerPort  TriggerType=function-call  VariantControl=Variant
      settings: CopyFcn=Stateflow.SLINSF.SimulinkMan.removeBlockPreDeleteFcn  PreDeleteFcn=Stateflow.SLINSF.SimulinkMan.unsafePredeleteFcn
  Abs : Abs
  Abs1 : Abs
  Divide : Product  Inputs=*/
  temptStop : Outport
  wires:
  accelRel/1 -> Abs1/1
  Divide/1 -> temptStop/1
  Abs1/1 -> Divide/2
  Abs/1 -> Divide/1
  velRel/1 -> Abs/1

== system TosAeb/FcwAebJa_Step_sys/ALG_AEBSystem/ALG_AEBController/AEB_LongTgtInfoCalc/LongObjCalcInfoCalc/AEBS_LongCollisionAssesApp/collisionSeverityHighCalc/collisionSeverityHighCalc1/BrkSysAtribution/FCWSnvtyEarly ==
  ObjectMovingState : Inport
  subjVel : Inport  Port=2
  subjLongAccel : Inport  Port=3
  Action Port : ActionPort  ActionPortLabel=case [ HMISettingEarly ]:
  Bus Creator : BusCreator  Inputs=3
  Bus Creator1 : BusCreator  Inputs=3
  Bus Creator2 : BusCreator  Inputs=2
  Compare To Constant : simulink/Logic and Bit Operations/Compare To Constant  relop===  const=AEB_ObjectMovingState.Still  OutDataTypeStr=boolean
  Compare To Constant1 : simulink/Logic and Bit Operations/Compare To Constant  relop===  const=AEB_ObjectMovingState.Still  OutDataTypeStr=boolean
  Compare To Constant2 : simulink/Logic and Bit Operations/Compare To Constant  relop===  const=AEB_ObjectMovingState.Still  OutDataTypeStr=boolean
  Constant : Constant  Commented=on  Value=P_AEB_LongCA_SeverityHighDlyTimeConfig
  DelayTimeHigh : Constant  Commented=on  Value=P_AEB_LongCA_SeverityHighBrkDlyTimeHighHmiEarly  OutDataTypeStr=single
  DelayTimeLow : Constant  Commented=on  Value=P_AEB_LongCA_SeverityHighBrkDlyTimeLowHmiEarly  OutDataTypeStr=single
  SubjAccelThres : simulink/Logic and Bit Operations/Compare To Constant  relop=<  const=P_AEB_LongCA_SeverityHighSubjAccelThes4BrkDlyTimeHmiEarly  OutDataTypeStr=boolean
  Switch{,2..4} (4 alike) : Switch  Criteria=u2 ~= 0
  Switch1 : Switch  Commented=on  Criteria=u2 ~= 0
  VelMaxReudceMO : Constant  Value=P_AEB_LongCA_SeverityHighVelMaxReduceMOHmiEarly
  VelMaxReudceSO : Constant  Value=P_AEB_LongCA_SeverityHighVelMaxReduceSOHmiEarly
  accelGradientMOLookUp : Lookup_n-D  NumberOfTableDimensions=1  BreakpointsForDimension1=P_AEB_LongCA_SeverityHighAccelGredientMOXHmiEarly  BeginIndexSearchUsingPreviousIndexResult=on  Table=P_AEB_LongCA_SeverityHighAccelGredientMOYHmiEarly  ExtrapMethod=Clip  InternalRulePriority=Speed  RndMeth=Simplest  InputSameDT=on
  accelGradientSOLookUp : Lookup_n-D  NumberOfTableDimensions=1  BreakpointsForDimension1=P_AEB_LongCA_SeverityHighAccelGredientSOXHmiEarly  BeginIndexSearchUsingPreviousIndexResult=on  Table=P_AEB_LongCA_SeverityHighAccelGredientSOYHmiEarly  ExtrapMethod=Clip  InternalRulePriority=Speed  RndMeth=Simplest  InputSameDT=on
  accelMaxReudceMOLookUp : Lookup_n-D  NumberOfTableDimensions=1  BreakpointsForDimension1=P_AEB_LongCA_SeverityHighAccelMaxDecMOXHmiEarly  BeginIndexSearchUsingPreviousIndexResult=on  Table=P_AEB_LongCA_SeverityHighAccelMaxDecMOYHmiEarly  ExtrapMethod=Clip  InternalRulePriority=Speed  RndMeth=Simplest  InputSameDT=on
  accelMaxReudceSOLookUp : Lookup_n-D  NumberOfTableDimensions=1  BreakpointsForDimension1=P_AEB_LongCA_SeverityHighAccelMaxDecSOXHmiEarly  BeginIndexSearchUsingPreviousIndexResult=on  Table=P_AEB_LongCA_SeverityHighAccelMaxDecSOYHmiEarly  ExtrapMethod=Clip  InternalRulePriority=Speed  RndMeth=Simplest  InputSameDT=on
  tBrakeLatencyLowMOLookUp : Lookup_n-D  NumberOfTableDimensions=1  BreakpointsForDimension1=P_AEB_LongCA_SeverityHighDlyTimeLowMOXHmiEarly  BeginIndexSearchUsingPreviousIndexResult=on  Table=P_AEB_LongCA_SeverityHighDlyTimeLowMOYHmiEarly  ExtrapMethod=Clip  InternalRulePriority=Speed  RndMeth=Simplest  InputSameDT=on
  tBrakeLatencyLowSOLookUp : Lookup_n-D  NumberOfTableDimensions=1  BreakpointsForDimension1=P_AEB_LongCA_SeverityHighDlyTimeLowSOXHmiEarly  BeginIndexSearchUsingPreviousIndexResult=on  Table=P_AEB_LongCA_SeverityHighDlyTimeLowSOYHmiEarly  ExtrapMethod=Clip  InternalRulePriority=Speed  RndMeth=Simplest  InputSameDT=on
  tBrakeLatencyMOLookUp : Lookup_n-D  NumberOfTableDimensions=1  BreakpointsForDimension1=P_AEB_LongCA_SeverityHighDlyTimeMOXHmiEarly  BeginIndexSearchUsingPreviousIndexResult=on  Table=P_AEB_LongCA_SeverityHighDlyTimeMOYHmiEarly  ExtrapMethod=Clip  InternalRulePriority=Speed  RndMeth=Simplest  InputSameDT=on
  tBrakeLatencySOLookUp : Lookup_n-D  NumberOfTableDimensions=1  BreakpointsForDimension1=P_AEB_LongCA_SeverityHighDlyTimeSOXHmiEarly  BeginIndexSearchUsingPreviousIndexResult=on  Table=P_AEB_LongCA_SeverityHighDlyTimeSOYHmiEarly  ExtrapMethod=Clip  InternalRulePriority=Speed  RndMeth=Simplest  InputSameDT=on
  brkSys : Outport
  wires:
  accelMaxReudceSOLookUp/1 -> Bus Creator/2  "accelMaxReudce"
  ObjectMovingState/1 -> [ObjectMovingState], Compare To Constant/1
  Bus Creator1/1 -> Switch/3
  accelGradientSOLookUp/1 -> Bus Creator/3  "accelGradient"
  subjLongAccel/1 -> SubjAccelThres/1
  Switch3/1 -> Bus Creator2/2  "tBrakeLatency"
  Switch/1 -> Bus Creator2/1  "brkSysAtribution"
  Bus Creator/1 -> Switch/1
  VelMaxReudceSO/1 -> Bus Creator/1  "velMaxReudce"
  Compare To Constant/1 -> Switch/2
  VelMaxReudceMO/1 -> Bus Creator1/1  "velMaxReudce"
  accelGradientMOLookUp/1 -> Bus Creator1/3  "accelGradient"
  accelMaxReudceMOLookUp/1 -> Bus Creator1/2  "accelMaxReudce"
  Bus Creator2/1 -> brkSys/1
  [ObjectMovingState] -> Compare To Constant1/1
  tBrakeLatencyMOLookUp/1 -> Switch2/3
  Compare To Constant1/1 -> Switch2/2
  tBrakeLatencySOLookUp/1 -> Switch2/1
  Switch2/1 -> Switch3/3  "tBrakeLatency"
  SubjAccelThres/1 -> Switch3/2
  tBrakeLatencyLowSOLookUp/1 -> Switch4/1
  Compare To Constant2/1 -> Switch4/2
  subjVel/1 -> tBrakeLatencyLowSOLookUp/1, tBrakeLatencyLowMOLookUp/1, tBrakeLatencyMOLookUp/1, tBrakeLatencySOLookUp/1, accelGradientMOLookUp/1, accelMaxReudceMOLookUp/1, accelGradientSOLookUp/1, accelMaxReudceSOLookUp/1
  tBrakeLatencyLowMOLookUp/1 -> Switch4/3
  [ObjectMovingState] -> Compare To Constant2/1
  Switch4/1 -> Switch3/1  "tBrakeLatency"
